simulink model "bee2_calib_corr"  (Simulink 7.1)
settings: Solver=ode45  StartTime=0.0  StopTime=100  RelTol=1e-3
undefined symbols (not defined in the file or in its folder): BEE2_usr
libraries: xbsIndex_r4 x2291, quant_xcorr_lib x1792, xps_library x56, xbsReplacements_r4 x32, casper_library_misc x2
(part 9 of 30)

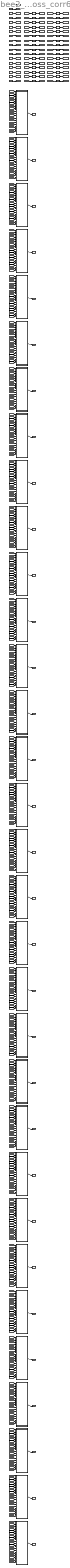
== system bee2_calib_corr/quant_cross_corr6 ==
  rst : Inport  OutDataType=sfix(16)  OutScaling=2^0
  en : Inport  Port=2  OutDataType=sfix(16)  OutScaling=2^0
  in1_16 : Inport  Port=3  OutDataType=sfix(16)  OutScaling=2^0
  in1_17 : Inport  Port=4  OutDataType=sfix(16)  OutScaling=2^0
  in1_18 : Inport  Port=5  OutDataType=sfix(16)  OutScaling=2^0
  in1_19 : Inport  Port=6  OutDataType=sfix(16)  OutScaling=2^0
  in1_20 : Inport  Port=7  OutDataType=sfix(16)  OutScaling=2^0
  in1_21 : Inport  Port=8  OutDataType=sfix(16)  OutScaling=2^0
  in1_22 : Inport  Port=9  OutDataType=sfix(16)  OutScaling=2^0
  in1_23 : Inport  Port=10  OutDataType=sfix(16)  OutScaling=2^0
  in2_16 : Inport  Port=11  OutDataType=sfix(16)  OutScaling=2^0
  in2_17 : Inport  Port=12  OutDataType=sfix(16)  OutScaling=2^0
  in2_18 : Inport  Port=13  OutDataType=sfix(16)  OutScaling=2^0
  in2_19 : Inport  Port=14  OutDataType=sfix(16)  OutScaling=2^0
  in2_20 : Inport  Port=15  OutDataType=sfix(16)  OutScaling=2^0
  in2_21 : Inport  Port=16  OutDataType=sfix(16)  OutScaling=2^0
  in2_22 : Inport  Port=17  OutDataType=sfix(16)  OutScaling=2^0
  in2_23 : Inport  Port=18  OutDataType=sfix(16)  OutScaling=2^0
  in1_16_del1 : xbsIndex_r4/Delay  en=off  latency=1  dbl_ovrd=off  reg_retiming=off  block_type=delay
      settings: xl_area=[0, 0, 0, 0, 0, 0, 0]
  in1_16_del2 : xbsIndex_r4/Delay  en=off  latency=1  dbl_ovrd=off  reg_retiming=off  block_type=delay
      settings: xl_area=[0, 0, 0, 0, 0, 0, 0]
  in1_17_del1 : xbsIndex_r4/Delay  en=off  latency=1  dbl_ovrd=off  reg_retiming=off  block_type=delay
      settings: xl_area=[0, 0, 0, 0, 0, 0, 0]
  in1_17_del2 : xbsIndex_r4/Delay  en=off  latency=1  dbl_ovrd=off  reg_retiming=off  block_type=delay
      settings: xl_area=[0, 0, 0, 0, 0, 0, 0]
  in1_18_del1 : xbsIndex_r4/Delay  en=off  latency=1  dbl_ovrd=off  reg_retiming=off  block_type=delay
      settings: xl_area=[0, 0, 0, 0, 0, 0, 0]
  in1_18_del2 : xbsIndex_r4/Delay  en=off  latency=1  dbl_ovrd=off  reg_retiming=off  block_type=delay
      settings: xl_area=[0, 0, 0, 0, 0, 0, 0]
  in1_19_del1 : xbsIndex_r4/Delay  en=off  latency=1  dbl_ovrd=off  reg_retiming=off  block_type=delay
      settings: xl_area=[0, 0, 0, 0, 0, 0, 0]
  in1_19_del2 : xbsIndex_r4/Delay  en=off  latency=1  dbl_ovrd=off  reg_retiming=off  block_type=delay
      settings: xl_area=[0, 0, 0, 0, 0, 0, 0]
  in1_20_del1 : xbsIndex_r4/Delay  en=off  latency=1  dbl_ovrd=off  reg_retiming=off  block_type=delay
      settings: xl_area=[0, 0, 0, 0, 0, 0, 0]
  in1_20_del2 : xbsIndex_r4/Delay  en=off  latency=1  dbl_ovrd=off  reg_retiming=off  block_type=delay
      settings: xl_area=[0, 0, 0, 0, 0, 0, 0]
  in1_21_del1 : xbsIndex_r4/Delay  en=off  latency=1  dbl_ovrd=off  reg_retiming=off  block_type=delay
      settings: xl_area=[0, 0, 0, 0, 0, 0, 0]
  in1_21_del2 : xbsIndex_r4/Delay  en=off  latency=1  dbl_ovrd=off  reg_retiming=off  block_type=delay
      settings: xl_area=[0, 0, 0, 0, 0, 0, 0]
  in1_22_del1 : xbsIndex_r4/Delay  en=off  latency=1  dbl_ovrd=off  reg_retiming=off  block_type=delay
      settings: xl_area=[0, 0, 0, 0, 0, 0, 0]
  in1_22_del2 : xbsIndex_r4/Delay  en=off  latency=1  dbl_ovrd=off  reg_retiming=off  block_type=delay
      settings: xl_area=[0, 0, 0, 0, 0, 0, 0]
  in1_23_del1 : xbsIndex_r4/Delay  en=off  latency=1  dbl_ovrd=off  reg_retiming=off  block_type=delay
      settings: xl_area=[0, 0, 0, 0, 0, 0, 0]
  in1_23_del2 : xbsIndex_r4/Delay  en=off  latency=1  dbl_ovrd=off  reg_retiming=off  block_type=delay
      settings: xl_area=[0, 0, 0, 0, 0, 0, 0]
  in2_16_del1 : xbsIndex_r4/Delay  en=off  latency=1  dbl_ovrd=off  reg_retiming=off  block_type=delay
      settings: xl_area=[0, 0, 0, 0, 0, 0, 0]
  in2_16_del2 : xbsIndex_r4/Delay  en=off  latency=1  dbl_ovrd=off  reg_retiming=off  block_type=delay
      settings: xl_area=[0, 0, 0, 0, 0, 0, 0]
  in2_17_del1 : xbsIndex_r4/Delay  en=off  latency=1  dbl_ovrd=off  reg_retiming=off  block_type=delay
      settings: xl_area=[0, 0, 0, 0, 0, 0, 0]
  in2_17_del2 : xbsIndex_r4/Delay  en=off  latency=1  dbl_ovrd=off  reg_retiming=off  block_type=delay
      settings: xl_area=[0, 0, 0, 0, 0, 0, 0]
  in2_18_del1 : xbsIndex_r4/Delay  en=off  latency=1  dbl_ovrd=off  reg_retiming=off  block_type=delay
      settings: xl_area=[0, 0, 0, 0, 0, 0, 0]
  in2_18_del2 : xbsIndex_r4/Delay  en=off  latency=1  dbl_ovrd=off  reg_retiming=off  block_type=delay
      settings: xl_area=[0, 0, 0, 0, 0, 0, 0]
  in2_19_del1 : xbsIndex_r4/Delay  en=off  latency=1  dbl_ovrd=off  reg_retiming=off  block_type=delay
      settings: xl_area=[0, 0, 0, 0, 0, 0, 0]
  in2_19_del2 : xbsIndex_r4/Delay  en=off  latency=1  dbl_ovrd=off  reg_retiming=off  block_type=delay
      settings: xl_area=[0, 0, 0, 0, 0, 0, 0]
  in2_20_del1 : xbsIndex_r4/Delay  en=off  latency=1  dbl_ovrd=off  reg_retiming=off  block_type=delay
      settings: xl_area=[0, 0, 0, 0, 0, 0, 0]
  in2_20_del2 : xbsIndex_r4/Delay  en=off  latency=1  dbl_ovrd=off  reg_retiming=off  block_type=delay
      settings: xl_area=[0, 0, 0, 0, 0, 0, 0]
  in2_21_del1 : xbsIndex_r4/Delay  en=off  latency=1  dbl_ovrd=off  reg_retiming=off  block_type=delay
      settings: xl_area=[0, 0, 0, 0, 0, 0, 0]
  in2_21_del2 : xbsIndex_r4/Delay  en=off  latency=1  dbl_ovrd=off  reg_retiming=off  block_type=delay
      settings: xl_area=[0, 0, 0, 0, 0, 0, 0]
  in2_22_del1 : xbsIndex_r4/Delay  en=off  latency=1  dbl_ovrd=off  reg_retiming=off  block_type=delay
      settings: xl_area=[0, 0, 0, 0, 0, 0, 0]
  in2_22_del2 : xbsIndex_r4/Delay  en=off  latency=1  dbl_ovrd=off  reg_retiming=off  block_type=delay
      settings: xl_area=[0, 0, 0, 0, 0, 0, 0]
  in2_23_del1 : xbsIndex_r4/Delay  en=off  latency=1  dbl_ovrd=off  reg_retiming=off  block_type=delay
      settings: xl_area=[0, 0, 0, 0, 0, 0, 0]
  in2_23_del2 : xbsIndex_r4/Delay  en=off  latency=1  dbl_ovrd=off  reg_retiming=off  block_type=delay
      settings: xl_area=[0, 0, 0, 0, 0, 0, 0]
  lag-1 : Subsystem  AncestorBlock=quant_xcorr_lib/quant_lag_element  mask(quant_lag_element){demux=3, lag=-1}
      mask prompts: demux "Demux (2^?):"; lag "Lag Number:"
      mask init:
      | quant_lag_element_init(gcb,...
      |     'demux', demux, 'lag', lag);
      settings: AttributesFormatString=demux=8, lag=-1
  lag-10 : Subsystem  AncestorBlock=quant_xcorr_lib/quant_lag_element  mask(quant_lag_element){demux=3, lag=-10}
      mask prompts: demux "Demux (2^?):"; lag "Lag Number:"
      mask init:
      | quant_lag_element_init(gcb,...
      |     'demux', demux, 'lag', lag);
      settings: AttributesFormatString=demux=8, lag=-10
  lag-11 : Subsystem  AncestorBlock=quant_xcorr_lib/quant_lag_element  mask(quant_lag_element){demux=3, lag=-11}
      mask prompts: demux "Demux (2^?):"; lag "Lag Number:"
      mask init:
      | quant_lag_element_init(gcb,...
      |     'demux', demux, 'lag', lag);
      settings: AttributesFormatString=demux=8, lag=-11
  lag-12 : Subsystem  AncestorBlock=quant_xcorr_lib/quant_lag_element  mask(quant_lag_element){demux=3, lag=-12}
      mask prompts: demux "Demux (2^?):"; lag "Lag Number:"
      mask init:
      | quant_lag_element_init(gcb,...
      |     'demux', demux, 'lag', lag);
      settings: AttributesFormatString=demux=8, lag=-12
  lag-13 : Subsystem  AncestorBlock=quant_xcorr_lib/quant_lag_element  mask(quant_lag_element){demux=3, lag=-13}
      mask prompts: demux "Demux (2^?):"; lag "Lag Number:"
      mask init:
      | quant_lag_element_init(gcb,...
      |     'demux', demux, 'lag', lag);
      settings: AttributesFormatString=demux=8, lag=-13
  lag-14 : Subsystem  AncestorBlock=quant_xcorr_lib/quant_lag_element  mask(quant_lag_element){demux=3, lag=-14}
      mask prompts: demux "Demux (2^?):"; lag "Lag Number:"
      mask init:
      | quant_lag_element_init(gcb,...
      |     'demux', demux, 'lag', lag);
      settings: AttributesFormatString=demux=8, lag=-14
  lag-15 : Subsystem  AncestorBlock=quant_xcorr_lib/quant_lag_element  mask(quant_lag_element){demux=3, lag=-15}
      mask prompts: demux "Demux (2^?):"; lag "Lag Number:"
      mask init:
      | quant_lag_element_init(gcb,...
      |     'demux', demux, 'lag', lag);
      settings: AttributesFormatString=demux=8, lag=-15
  lag-16 : Subsystem  AncestorBlock=quant_xcorr_lib/quant_lag_element  mask(quant_lag_element){demux=3, lag=-16}
      mask prompts: demux "Demux (2^?):"; lag "Lag Number:"
      mask init:
      | quant_lag_element_init(gcb,...
      |     'demux', demux, 'lag', lag);
      settings: AttributesFormatString=demux=8, lag=-16
  lag-2 : Subsystem  AncestorBlock=quant_xcorr_lib/quant_lag_element  mask(quant_lag_element){demux=3, lag=-2}
      mask prompts: demux "Demux (2^?):"; lag "Lag Number:"
      mask init:
      | quant_lag_element_init(gcb,...
      |     'demux', demux, 'lag', lag);
      settings: AttributesFormatString=demux=8, lag=-2
  lag-3 : Subsystem  AncestorBlock=quant_xcorr_lib/quant_lag_element  mask(quant_lag_element){demux=3, lag=-3}
      mask prompts: demux "Demux (2^?):"; lag "Lag Number:"
      mask init:
      | quant_lag_element_init(gcb,...
      |     'demux', demux, 'lag', lag);
      settings: AttributesFormatString=demux=8, lag=-3
  lag-4 : Subsystem  AncestorBlock=quant_xcorr_lib/quant_lag_element  mask(quant_lag_element){demux=3, lag=-4}
      mask prompts: demux "Demux (2^?):"; lag "Lag Number:"
      mask init:
      | quant_lag_element_init(gcb,...
      |     'demux', demux, 'lag', lag);
      settings: AttributesFormatString=demux=8, lag=-4
  lag-5 : Subsystem  AncestorBlock=quant_xcorr_lib/quant_lag_element  mask(quant_lag_element){demux=3, lag=-5}
      mask prompts: demux "Demux (2^?):"; lag "Lag Number:"
      mask init:
      | quant_lag_element_init(gcb,...
      |     'demux', demux, 'lag', lag);
      settings: AttributesFormatString=demux=8, lag=-5
  lag-6 : Subsystem  AncestorBlock=quant_xcorr_lib/quant_lag_element  mask(quant_lag_element){demux=3, lag=-6}
      mask prompts: demux "Demux (2^?):"; lag "Lag Number:"
      mask init:
      | quant_lag_element_init(gcb,...
      |     'demux', demux, 'lag', lag);
      settings: AttributesFormatString=demux=8, lag=-6
  lag-7 : Subsystem  AncestorBlock=quant_xcorr_lib/quant_lag_element  mask(quant_lag_element){demux=3, lag=-7}
      mask prompts: demux "Demux (2^?):"; lag "Lag Number:"
      mask init:
      | quant_lag_element_init(gcb,...
      |     'demux', demux, 'lag', lag);
      settings: AttributesFormatString=demux=8, lag=-7
  lag-8 : Subsystem  AncestorBlock=quant_xcorr_lib/quant_lag_element  mask(quant_lag_element){demux=3, lag=-8}
      mask prompts: demux "Demux (2^?):"; lag "Lag Number:"
      mask init:
      | quant_lag_element_init(gcb,...
      |     'demux', demux, 'lag', lag);
      settings: AttributesFormatString=demux=8, lag=-8
  lag-9 : Subsystem  AncestorBlock=quant_xcorr_lib/quant_lag_element  mask(quant_lag_element){demux=3, lag=-9}
      mask prompts: demux "Demux (2^?):"; lag "Lag Number:"
      mask init:
      | quant_lag_element_init(gcb,...
      |     'demux', demux, 'lag', lag);
      settings: AttributesFormatString=demux=8, lag=-9
  lag0 : Subsystem  AncestorBlock=quant_xcorr_lib/quant_lag_element  mask(quant_lag_element){demux=3, lag=0}
      mask prompts: demux "Demux (2^?):"; lag "Lag Number:"
      mask init:
      | quant_lag_element_init(gcb,...
      |     'demux', demux, 'lag', lag);
      settings: AttributesFormatString=demux=8, lag=0
  lag1 : Subsystem  AncestorBlock=quant_xcorr_lib/quant_lag_element  mask(quant_lag_element){demux=3, lag=1}
      mask prompts: demux "Demux (2^?):"; lag "Lag Number:"
      mask init:
      | quant_lag_element_init(gcb,...
      |     'demux', demux, 'lag', lag);
      settings: AttributesFormatString=demux=8, lag=1
  lag10 : Subsystem  AncestorBlock=quant_xcorr_lib/quant_lag_element  mask(quant_lag_element){demux=3, lag=10}
      mask prompts: demux "Demux (2^?):"; lag "Lag Number:"
      mask init:
      | quant_lag_element_init(gcb,...
      |     'demux', demux, 'lag', lag);
      settings: AttributesFormatString=demux=8, lag=10
  lag11 : Subsystem  AncestorBlock=quant_xcorr_lib/quant_lag_element  mask(quant_lag_element){demux=3, lag=11}
      mask prompts: demux "Demux (2^?):"; lag "Lag Number:"
      mask init:
      | quant_lag_element_init(gcb,...
      |     'demux', demux, 'lag', lag);
      settings: AttributesFormatString=demux=8, lag=11
  lag12 : Subsystem  AncestorBlock=quant_xcorr_lib/quant_lag_element  mask(quant_lag_element){demux=3, lag=12}
      mask prompts: demux "Demux (2^?):"; lag "Lag Number:"
      mask init:
      | quant_lag_element_init(gcb,...
      |     'demux', demux, 'lag', lag);
      settings: AttributesFormatString=demux=8, lag=12
  lag13 : Subsystem  AncestorBlock=quant_xcorr_lib/quant_lag_element  mask(quant_lag_element){demux=3, lag=13}
      mask prompts: demux "Demux (2^?):"; lag "Lag Number:"
      mask init:
      | quant_lag_element_init(gcb,...
      |     'demux', demux, 'lag', lag);
      settings: AttributesFormatString=demux=8, lag=13
  lag14 : Subsystem  AncestorBlock=quant_xcorr_lib/quant_lag_element  mask(quant_lag_element){demux=3, lag=14}
      mask prompts: demux "Demux (2^?):"; lag "Lag Number:"
      mask init:
      | quant_lag_element_init(gcb,...
      |     'demux', demux, 'lag', lag);
      settings: AttributesFormatString=demux=8, lag=14
  lag15 : Subsystem  AncestorBlock=quant_xcorr_lib/quant_lag_element  mask(quant_lag_element){demux=3, lag=15}
      mask prompts: demux "Demux (2^?):"; lag "Lag Number:"
      mask init:
      | quant_lag_element_init(gcb,...
      |     'demux', demux, 'lag', lag);
      settings: AttributesFormatString=demux=8, lag=15
  lag2 : Subsystem  AncestorBlock=quant_xcorr_lib/quant_lag_element  mask(quant_lag_element){demux=3, lag=2}
      mask prompts: demux "Demux (2^?):"; lag "Lag Number:"
      mask init:
      | quant_lag_element_init(gcb,...
      |     'demux', demux, 'lag', lag);
      settings: AttributesFormatString=demux=8, lag=2
  lag3 : Subsystem  AncestorBlock=quant_xcorr_lib/quant_lag_element  mask(quant_lag_element){demux=3, lag=3}
      mask prompts: demux "Demux (2^?):"; lag "Lag Number:"
      mask init:
      | quant_lag_element_init(gcb,...
      |     'demux', demux, 'lag', lag);
      settings: AttributesFormatString=demux=8, lag=3
  lag4 : Subsystem  AncestorBlock=quant_xcorr_lib/quant_lag_element  mask(quant_lag_element){demux=3, lag=4}
      mask prompts: demux "Demux (2^?):"; lag "Lag Number:"
      mask init:
      | quant_lag_element_init(gcb,...
      |     'demux', demux, 'lag', lag);
      settings: AttributesFormatString=demux=8, lag=4
  lag5 : Subsystem  AncestorBlock=quant_xcorr_lib/quant_lag_element  mask(quant_lag_element){demux=3, lag=5}
      mask prompts: demux "Demux (2^?):"; lag "Lag Number:"
      mask init:
      | quant_lag_element_init(gcb,...
      |     'demux', demux, 'lag', lag);
      settings: AttributesFormatString=demux=8, lag=5
  lag6 : Subsystem  AncestorBlock=quant_xcorr_lib/quant_lag_element  mask(quant_lag_element){demux=3, lag=6}
      mask prompts: demux "Demux (2^?):"; lag "Lag Number:"
      mask init:
      | quant_lag_element_init(gcb,...
      |     'demux', demux, 'lag', lag);
      settings: AttributesFormatString=demux=8, lag=6
  lag7 : Subsystem  AncestorBlock=quant_xcorr_lib/quant_lag_element  mask(quant_lag_element){demux=3, lag=7}
      mask prompts: demux "Demux (2^?):"; lag "Lag Number:"
      mask init:
      | quant_lag_element_init(gcb,...
      |     'demux', demux, 'lag', lag);
      settings: AttributesFormatString=demux=8, lag=7
  lag8 : Subsystem  AncestorBlock=quant_xcorr_lib/quant_lag_element  mask(quant_lag_element){demux=3, lag=8}
      mask prompts: demux "Demux (2^?):"; lag "Lag Number:"
      mask init:
      | quant_lag_element_init(gcb,...
      |     'demux', demux, 'lag', lag);
      settings: AttributesFormatString=demux=8, lag=8
  lag9 : Subsystem  AncestorBlock=quant_xcorr_lib/quant_lag_element  mask(quant_lag_element){demux=3, lag=9}
      mask prompts: demux "Demux (2^?):"; lag "Lag Number:"
      mask init:
      | quant_lag_element_init(gcb,...
      |     'demux', demux, 'lag', lag);
      settings: AttributesFormatString=demux=8, lag=9
  out-16 : Outport  OutDataType=sfix(16)  OutScaling=2^0
  out-15 : Outport  Port=2  OutDataType=sfix(16)  OutScaling=2^0
  out-14 : Outport  Port=3  OutDataType=sfix(16)  OutScaling=2^0
  out-13 : Outport  Port=4  OutDataType=sfix(16)  OutScaling=2^0
  out-12 : Outport  Port=5  OutDataType=sfix(16)  OutScaling=2^0
  out-11 : Outport  Port=6  OutDataType=sfix(16)  OutScaling=2^0
  out-10 : Outport  Port=7  OutDataType=sfix(16)  OutScaling=2^0
  out-9 : Outport  Port=8  OutDataType=sfix(16)  OutScaling=2^0
  out-8 : Outport  Port=9  OutDataType=sfix(16)  OutScaling=2^0
  out-7 : Outport  Port=10  OutDataType=sfix(16)  OutScaling=2^0
  out-6 : Outport  Port=11  OutDataType=sfix(16)  OutScaling=2^0
  out-5 : Outport  Port=12  OutDataType=sfix(16)  OutScaling=2^0
  out-4 : Outport  Port=13  OutDataType=sfix(16)  OutScaling=2^0
  out-3 : Outport  Port=14  OutDataType=sfix(16)  OutScaling=2^0
  out-2 : Outport  Port=15  OutDataType=sfix(16)  OutScaling=2^0
  out-1 : Outport  Port=16  OutDataType=sfix(16)  OutScaling=2^0
  out0 : Outport  Port=17  OutDataType=sfix(16)  OutScaling=2^0
  out1 : Outport  Port=18  OutDataType=sfix(16)  OutScaling=2^0
  out2 : Outport  Port=19  OutDataType=sfix(16)  OutScaling=2^0
  out3 : Outport  Port=20  OutDataType=sfix(16)  OutScaling=2^0
  out4 : Outport  Port=21  OutDataType=sfix(16)  OutScaling=2^0
  out5 : Outport  Port=22  OutDataType=sfix(16)  OutScaling=2^0
  out6 : Outport  Port=23  OutDataType=sfix(16)  OutScaling=2^0
  out7 : Outport  Port=24  OutDataType=sfix(16)  OutScaling=2^0
  out8 : Outport  Port=25  OutDataType=sfix(16)  OutScaling=2^0
  out9 : Outport  Port=26  OutDataType=sfix(16)  OutScaling=2^0
  out10 : Outport  Port=27  OutDataType=sfix(16)  OutScaling=2^0
  out11 : Outport  Port=28  OutDataType=sfix(16)  OutScaling=2^0
  out12 : Outport  Port=29  OutDataType=sfix(16)  OutScaling=2^0
  out13 : Outport  Port=30  OutDataType=sfix(16)  OutScaling=2^0
  out14 : Outport  Port=31  OutDataType=sfix(16)  OutScaling=2^0
  out15 : Outport  Port=32  OutDataType=sfix(16)  OutScaling=2^0
  wires:
  rst/1 -> [reset]
  en/1 -> [enable]
  in1_16/1 -> [in1_16]
  [in1_16] -> in1_16_del1/1
  in1_16_del1/1 -> [in1_8]
  [in1_8] -> in1_16_del2/1
  in1_16_del2/1 -> [in1_0]
  in1_17/1 -> [in1_17]
  [in1_17] -> in1_17_del1/1
  in1_17_del1/1 -> [in1_9]
  [in1_9] -> in1_17_del2/1
  in1_17_del2/1 -> [in1_1]
  in1_18/1 -> [in1_18]
  [in1_18] -> in1_18_del1/1
  in1_18_del1/1 -> [in1_10]
  [in1_10] -> in1_18_del2/1
  in1_18_del2/1 -> [in1_2]
  in1_19/1 -> [in1_19]
  [in1_19] -> in1_19_del1/1
  in1_19_del1/1 -> [in1_11]
  [in1_11] -> in1_19_del2/1
  in1_19_del2/1 -> [in1_3]
  in1_20/1 -> [in1_20]
  [in1_20] -> in1_20_del1/1
  in1_20_del1/1 -> [in1_12]
  [in1_12] -> in1_20_del2/1
  in1_20_del2/1 -> [in1_4]
  in1_21/1 -> [in1_21]
  [in1_21] -> in1_21_del1/1
  in1_21_del1/1 -> [in1_13]
  [in1_13] -> in1_21_del2/1
  in1_21_del2/1 -> [in1_5]
  in1_22/1 -> [in1_22]
  [in1_22] -> in1_22_del1/1
  in1_22_del1/1 -> [in1_14]
  [in1_14] -> in1_22_del2/1
  in1_22_del2/1 -> [in1_6]
  in1_23/1 -> [in1_23]
  [in1_23] -> in1_23_del1/1
  in1_23_del1/1 -> [in1_15]
  [in1_15] -> in1_23_del2/1
  in1_23_del2/1 -> [in1_7]
  in2_16/1 -> [in2_16]
  [in2_16] -> in2_16_del1/1
  in2_16_del1/1 -> [in2_8]
  [in2_8] -> in2_16_del2/1
  in2_16_del2/1 -> [in2_0]
  in2_17/1 -> [in2_17]
  [in2_17] -> in2_17_del1/1
  in2_17_del1/1 -> [in2_9]
  [in2_9] -> in2_17_del2/1
  in2_17_del2/1 -> [in2_1]
  in2_18/1 -> [in2_18]
  [in2_18] -> in2_18_del1/1
  in2_18_del1/1 -> [in2_10]
  [in2_10] -> in2_18_del2/1
  in2_18_del2/1 -> [in2_2]
  in2_19/1 -> [in2_19]
  [in2_19] -> in2_19_del1/1
  in2_19_del1/1 -> [in2_11]
  [in2_11] -> in2_19_del2/1
  in2_19_del2/1 -> [in2_3]
  in2_20/1 -> [in2_20]
  [in2_20] -> in2_20_del1/1
  in2_20_del1/1 -> [in2_12]
  [in2_12] -> in2_20_del2/1
  in2_20_del2/1 -> [in2_4]
  in2_21/1 -> [in2_21]
  [in2_21] -> in2_21_del1/1
  in2_21_del1/1 -> [in2_13]
  [in2_13] -> in2_21_del2/1
  in2_21_del2/1 -> [in2_5]
  in2_22/1 -> [in2_22]
  [in2_22] -> in2_22_del1/1
  in2_22_del1/1 -> [in2_14]
  [in2_14] -> in2_22_del2/1
  in2_22_del2/1 -> [in2_6]
  in2_23/1 -> [in2_23]
  [in2_23] -> in2_23_del1/1
  in2_23_del1/1 -> [in2_15]
  [in2_15] -> in2_23_del2/1
  in2_23_del2/1 -> [in2_7]
  [in1_16] -> lag-16/1(in1_0)
  [in1_17] -> lag-16/2(in1_1)
  [in1_18] -> lag-16/3(in1_2)
  [in1_19] -> lag-16/4(in1_3)
  [in1_20] -> lag-16/5(in1_4)
  [in1_21] -> lag-16/6(in1_5)
  [in1_22] -> lag-16/7(in1_6)
  [in1_23] -> lag-16/8(in1_7)
  [in2_0] -> lag-16/9(in2_0)
  [in2_1] -> lag-16/10(in2_1)
  [in2_2] -> lag-16/11(in2_2)
  [in2_3] -> lag-16/12(in2_3)
  [in2_4] -> lag-16/13(in2_4)
  [in2_5] -> lag-16/14(in2_5)
  [in2_6] -> lag-16/15(in2_6)
  [in2_7] -> lag-16/16(in2_7)
  [reset] -> lag-16/17(rst)
  [enable] -> lag-16/18(en)
  lag-16/1(acc_out) -> out-16/1
  [in1_15] -> lag-15/1(in1_0)
  [in1_16] -> lag-15/2(in1_1)
  [in1_17] -> lag-15/3(in1_2)
  [in1_18] -> lag-15/4(in1_3)
  [in1_19] -> lag-15/5(in1_4)
  [in1_20] -> lag-15/6(in1_5)
  [in1_21] -> lag-15/7(in1_6)
  [in1_22] -> lag-15/8(in1_7)
  [in2_0] -> lag-15/9(in2_0)
  [in2_1] -> lag-15/10(in2_1)
  [in2_2] -> lag-15/11(in2_2)
  [in2_3] -> lag-15/12(in2_3)
  [in2_4] -> lag-15/13(in2_4)
  [in2_5] -> lag-15/14(in2_5)
  [in2_6] -> lag-15/15(in2_6)
  [in2_7] -> lag-15/16(in2_7)
  [reset] -> lag-15/17(rst)
  [enable] -> lag-15/18(en)
  lag-15/1(acc_out) -> out-15/1
  [in1_14] -> lag-14/1(in1_0)
  [in1_15] -> lag-14/2(in1_1)
  [in1_16] -> lag-14/3(in1_2)
  [in1_17] -> lag-14/4(in1_3)
  [in1_18] -> lag-14/5(in1_4)
  [in1_19] -> lag-14/6(in1_5)
  [in1_20] -> lag-14/7(in1_6)
  [in1_21] -> lag-14/8(in1_7)
  [in2_0] -> lag-14/9(in2_0)
  [in2_1] -> lag-14/10(in2_1)
  [in2_2] -> lag-14/11(in2_2)
  [in2_3] -> lag-14/12(in2_3)
  [in2_4] -> lag-14/13(in2_4)
  [in2_5] -> lag-14/14(in2_5)
  [in2_6] -> lag-14/15(in2_6)
  [in2_7] -> lag-14/16(in2_7)
  [reset] -> lag-14/17(rst)
  [enable] -> lag-14/18(en)
  lag-14/1(acc_out) -> out-14/1
  [in1_13] -> lag-13/1(in1_0)
  [in1_14] -> lag-13/2(in1_1)
  [in1_15] -> lag-13/3(in1_2)
  [in1_16] -> lag-13/4(in1_3)
  [in1_17] -> lag-13/5(in1_4)
  [in1_18] -> lag-13/6(in1_5)
  [in1_19] -> lag-13/7(in1_6)
  [in1_20] -> lag-13/8(in1_7)
  [in2_0] -> lag-13/9(in2_0)
  [in2_1] -> lag-13/10(in2_1)
  [in2_2] -> lag-13/11(in2_2)
  [in2_3] -> lag-13/12(in2_3)
  [in2_4] -> lag-13/13(in2_4)
  [in2_5] -> lag-13/14(in2_5)
  [in2_6] -> lag-13/15(in2_6)
  [in2_7] -> lag-13/16(in2_7)
  [reset] -> lag-13/17(rst)
  [enable] -> lag-13/18(en)
  lag-13/1(acc_out) -> out-13/1
  [in1_12] -> lag-12/1(in1_0)
  [in1_13] -> lag-12/2(in1_1)
  [in1_14] -> lag-12/3(in1_2)
  [in1_15] -> lag-12/4(in1_3)
  [in1_16] -> lag-12/5(in1_4)
  [in1_17] -> lag-12/6(in1_5)
  [in1_18] -> lag-12/7(in1_6)
  [in1_19] -> lag-12/8(in1_7)
  [in2_0] -> lag-12/9(in2_0)
  [in2_1] -> lag-12/10(in2_1)
  [in2_2] -> lag-12/11(in2_2)
  [in2_3] -> lag-12/12(in2_3)
  [in2_4] -> lag-12/13(in2_4)
  [in2_5] -> lag-12/14(in2_5)
  [in2_6] -> lag-12/15(in2_6)
  [in2_7] -> lag-12/16(in2_7)
  [reset] -> lag-12/17(rst)
  [enable] -> lag-12/18(en)
  lag-12/1(acc_out) -> out-12/1
  [in1_11] -> lag-11/1(in1_0)
  [in1_12] -> lag-11/2(in1_1)
  [in1_13] -> lag-11/3(in1_2)
  [in1_14] -> lag-11/4(in1_3)
  [in1_15] -> lag-11/5(in1_4)
  [in1_16] -> lag-11/6(in1_5)
  [in1_17] -> lag-11/7(in1_6)
  [in1_18] -> lag-11/8(in1_7)
  [in2_0] -> lag-11/9(in2_0)
  [in2_1] -> lag-11/10(in2_1)
  [in2_2] -> lag-11/11(in2_2)
  [in2_3] -> lag-11/12(in2_3)
  [in2_4] -> lag-11/13(in2_4)
  [in2_5] -> lag-11/14(in2_5)
  [in2_6] -> lag-11/15(in2_6)
  [in2_7] -> lag-11/16(in2_7)
  [reset] -> lag-11/17(rst)
  [enable] -> lag-11/18(en)
  lag-11/1(acc_out) -> out-11/1
  [in1_10] -> lag-10/1(in1_0)
  [in1_11] -> lag-10/2(in1_1)
  [in1_12] -> lag-10/3(in1_2)
  [in1_13] -> lag-10/4(in1_3)
  [in1_14] -> lag-10/5(in1_4)
  [in1_15] -> lag-10/6(in1_5)
  [in1_16] -> lag-10/7(in1_6)
  [in1_17] -> lag-10/8(in1_7)
  [in2_0] -> lag-10/9(in2_0)
  [in2_1] -> lag-10/10(in2_1)
  [in2_2] -> lag-10/11(in2_2)
  [in2_3] -> lag-10/12(in2_3)
  [in2_4] -> lag-10/13(in2_4)
  [in2_5] -> lag-10/14(in2_5)
  [in2_6] -> lag-10/15(in2_6)
  [in2_7] -> lag-10/16(in2_7)
  [reset] -> lag-10/17(rst)
  [enable] -> lag-10/18(en)
  lag-10/1(acc_out) -> out-10/1
  [in1_9] -> lag-9/1(in1_0)
  [in1_10] -> lag-9/2(in1_1)
  [in1_11] -> lag-9/3(in1_2)
  [in1_12] -> lag-9/4(in1_3)
  [in1_13] -> lag-9/5(in1_4)
  [in1_14] -> lag-9/6(in1_5)
  [in1_15] -> lag-9/7(in1_6)
  [in1_16] -> lag-9/8(in1_7)
  [in2_0] -> lag-9/9(in2_0)
  [in2_1] -> lag-9/10(in2_1)
  [in2_2] -> lag-9/11(in2_2)
  [in2_3] -> lag-9/12(in2_3)
  [in2_4] -> lag-9/13(in2_4)
  [in2_5] -> lag-9/14(in2_5)
  [in2_6] -> lag-9/15(in2_6)
  [in2_7] -> lag-9/16(in2_7)
  [reset] -> lag-9/17(rst)
  [enable] -> lag-9/18(en)
  lag-9/1(acc_out) -> out-9/1
  [in1_8] -> lag-8/1(in1_0)
  [in1_9] -> lag-8/2(in1_1)
  [in1_10] -> lag-8/3(in1_2)
  [in1_11] -> lag-8/4(in1_3)
  [in1_12] -> lag-8/5(in1_4)
  [in1_13] -> lag-8/6(in1_5)
  [in1_14] -> lag-8/7(in1_6)
  [in1_15] -> lag-8/8(in1_7)
  [in2_0] -> lag-8/9(in2_0)
  [in2_1] -> lag-8/10(in2_1)
  [in2_2] -> lag-8/11(in2_2)
  [in2_3] -> lag-8/12(in2_3)
  [in2_4] -> lag-8/13(in2_4)
  [in2_5] -> lag-8/14(in2_5)
  [in2_6] -> lag-8/15(in2_6)
  [in2_7] -> lag-8/16(in2_7)
  [reset] -> lag-8/17(rst)
  [enable] -> lag-8/18(en)
  lag-8/1(acc_out) -> out-8/1
  [in1_7] -> lag-7/1(in1_0)
  [in1_8] -> lag-7/2(in1_1)
  [in1_9] -> lag-7/3(in1_2)
  [in1_10] -> lag-7/4(in1_3)
  [in1_11] -> lag-7/5(in1_4)
  [in1_12] -> lag-7/6(in1_5)
  [in1_13] -> lag-7/7(in1_6)
  [in1_14] -> lag-7/8(in1_7)
  [in2_0] -> lag-7/9(in2_0)
  [in2_1] -> lag-7/10(in2_1)
  [in2_2] -> lag-7/11(in2_2)
  [in2_3] -> lag-7/12(in2_3)
  [in2_4] -> lag-7/13(in2_4)
  [in2_5] -> lag-7/14(in2_5)
  [in2_6] -> lag-7/15(in2_6)
  [in2_7] -> lag-7/16(in2_7)
  [reset] -> lag-7/17(rst)
  [enable] -> lag-7/18(en)
  lag-7/1(acc_out) -> out-7/1
  [in1_6] -> lag-6/1(in1_0)
  [in1_7] -> lag-6/2(in1_1)
  [in1_8] -> lag-6/3(in1_2)
  [in1_9] -> lag-6/4(in1_3)
  [in1_10] -> lag-6/5(in1_4)
  [in1_11] -> lag-6/6(in1_5)
  [in1_12] -> lag-6/7(in1_6)
  [in1_13] -> lag-6/8(in1_7)
  [in2_0] -> lag-6/9(in2_0)
  [in2_1] -> lag-6/10(in2_1)
  [in2_2] -> lag-6/11(in2_2)
  [in2_3] -> lag-6/12(in2_3)
  [in2_4] -> lag-6/13(in2_4)
  [in2_5] -> lag-6/14(in2_5)
  [in2_6] -> lag-6/15(in2_6)
  [in2_7] -> lag-6/16(in2_7)
  [reset] -> lag-6/17(rst)
  [enable] -> lag-6/18(en)
  lag-6/1(acc_out) -> out-6/1
  [in1_5] -> lag-5/1(in1_0)
  [in1_6] -> lag-5/2(in1_1)
  [in1_7] -> lag-5/3(in1_2)
  [in1_8] -> lag-5/4(in1_3)
  [in1_9] -> lag-5/5(in1_4)
  [in1_10] -> lag-5/6(in1_5)
  [in1_11] -> lag-5/7(in1_6)
  [in1_12] -> lag-5/8(in1_7)
  [in2_0] -> lag-5/9(in2_0)
  [in2_1] -> lag-5/10(in2_1)
  [in2_2] -> lag-5/11(in2_2)
  [in2_3] -> lag-5/12(in2_3)
  [in2_4] -> lag-5/13(in2_4)
  [in2_5] -> lag-5/14(in2_5)
  [in2_6] -> lag-5/15(in2_6)
  [in2_7] -> lag-5/16(in2_7)
  [reset] -> lag-5/17(rst)
  [enable] -> lag-5/18(en)
  lag-5/1(acc_out) -> out-5/1
  [in1_4] -> lag-4/1(in1_0)
  [in1_5] -> lag-4/2(in1_1)
  [in1_6] -> lag-4/3(in1_2)
  [in1_7] -> lag-4/4(in1_3)
  [in1_8] -> lag-4/5(in1_4)
  [in1_9] -> lag-4/6(in1_5)
  [in1_10] -> lag-4/7(in1_6)
  [in1_11] -> lag-4/8(in1_7)
  [in2_0] -> lag-4/9(in2_0)
  [in2_1] -> lag-4/10(in2_1)
  [in2_2] -> lag-4/11(in2_2)
  [in2_3] -> lag-4/12(in2_3)
  [in2_4] -> lag-4/13(in2_4)
  [in2_5] -> lag-4/14(in2_5)
  [in2_6] -> lag-4/15(in2_6)
  [in2_7] -> lag-4/16(in2_7)
  [reset] -> lag-4/17(rst)
  [enable] -> lag-4/18(en)
  lag-4/1(acc_out) -> out-4/1
  [in1_3] -> lag-3/1(in1_0)
  [in1_4] -> lag-3/2(in1_1)
  [in1_5] -> lag-3/3(in1_2)
  [in1_6] -> lag-3/4(in1_3)
  [in1_7] -> lag-3/5(in1_4)
  [in1_8] -> lag-3/6(in1_5)
  [in1_9] -> lag-3/7(in1_6)
  [in1_10] -> lag-3/8(in1_7)
  [in2_0] -> lag-3/9(in2_0)
  [in2_1] -> lag-3/10(in2_1)
  [in2_2] -> lag-3/11(in2_2)
  [in2_3] -> lag-3/12(in2_3)
  [in2_4] -> lag-3/13(in2_4)
  [in2_5] -> lag-3/14(in2_5)
  [in2_6] -> lag-3/15(in2_6)
  [in2_7] -> lag-3/16(in2_7)
  [reset] -> lag-3/17(rst)
  [enable] -> lag-3/18(en)
  lag-3/1(acc_out) -> out-3/1
  [in1_2] -> lag-2/1(in1_0)
  [in1_3] -> lag-2/2(in1_1)
  [in1_4] -> lag-2/3(in1_2)
  [in1_5] -> lag-2/4(in1_3)
  [in1_6] -> lag-2/5(in1_4)
  [in1_7] -> lag-2/6(in1_5)
  [in1_8] -> lag-2/7(in1_6)
  [in1_9] -> lag-2/8(in1_7)
  [in2_0] -> lag-2/9(in2_0)
  [in2_1] -> lag-2/10(in2_1)
  [in2_2] -> lag-2/11(in2_2)
  [in2_3] -> lag-2/12(in2_3)
  [in2_4] -> lag-2/13(in2_4)
  [in2_5] -> lag-2/14(in2_5)
  [in2_6] -> lag-2/15(in2_6)
  [in2_7] -> lag-2/16(in2_7)
  [reset] -> lag-2/17(rst)
  [enable] -> lag-2/18(en)
  lag-2/1(acc_out) -> out-2/1
  [in1_1] -> lag-1/1(in1_0)
  [in1_2] -> lag-1/2(in1_1)
  [in1_3] -> lag-1/3(in1_2)
  [in1_4] -> lag-1/4(in1_3)
  [in1_5] -> lag-1/5(in1_4)
  [in1_6] -> lag-1/6(in1_5)
  [in1_7] -> lag-1/7(in1_6)
  [in1_8] -> lag-1/8(in1_7)
  [in2_0] -> lag-1/9(in2_0)
  [in2_1] -> lag-1/10(in2_1)
  [in2_2] -> lag-1/11(in2_2)
  [in2_3] -> lag-1/12(in2_3)
  [in2_4] -> lag-1/13(in2_4)
  [in2_5] -> lag-1/14(in2_5)
  [in2_6] -> lag-1/15(in2_6)
  [in2_7] -> lag-1/16(in2_7)
  [reset] -> lag-1/17(rst)
  [enable] -> lag-1/18(en)
  lag-1/1(acc_out) -> out-1/1
  [in1_0] -> lag0/1(in1_0)
  [in1_1] -> lag0/2(in1_1)
  [in1_2] -> lag0/3(in1_2)
  [in1_3] -> lag0/4(in1_3)
  [in1_4] -> lag0/5(in1_4)
  [in1_5] -> lag0/6(in1_5)
  [in1_6] -> lag0/7(in1_6)
  [in1_7] -> lag0/8(in1_7)
  [in2_0] -> lag0/9(in2_0)
  [in2_1] -> lag0/10(in2_1)
  [in2_2] -> lag0/11(in2_2)
  [in2_3] -> lag0/12(in2_3)
  [in2_4] -> lag0/13(in2_4)
  [in2_5] -> lag0/14(in2_5)
  [in2_6] -> lag0/15(in2_6)
  [in2_7] -> lag0/16(in2_7)
  [reset] -> lag0/17(rst)
  [enable] -> lag0/18(en)
  lag0/1(acc_out) -> out0/1
  [in1_0] -> lag1/1(in1_0)
  [in1_1] -> lag1/2(in1_1)
  [in1_2] -> lag1/3(in1_2)
  [in1_3] -> lag1/4(in1_3)
  [in1_4] -> lag1/5(in1_4)
  [in1_5] -> lag1/6(in1_5)
  [in1_6] -> lag1/7(in1_6)
  [in1_7] -> lag1/8(in1_7)
  [in2_1] -> lag1/9(in2_0)
  [in2_2] -> lag1/10(in2_1)
  [in2_3] -> lag1/11(in2_2)
  [in2_4] -> lag1/12(in2_3)
  [in2_5] -> lag1/13(in2_4)
  [in2_6] -> lag1/14(in2_5)
  [in2_7] -> lag1/15(in2_6)
  [in2_8] -> lag1/16(in2_7)
  [reset] -> lag1/17(rst)
  [enable] -> lag1/18(en)
  lag1/1(acc_out) -> out1/1
  [in1_0] -> lag2/1(in1_0)
  [in1_1] -> lag2/2(in1_1)
  [in1_2] -> lag2/3(in1_2)
  [in1_3] -> lag2/4(in1_3)
  [in1_4] -> lag2/5(in1_4)
  [in1_5] -> lag2/6(in1_5)
  [in1_6] -> lag2/7(in1_6)
  [in1_7] -> lag2/8(in1_7)
  [in2_2] -> lag2/9(in2_0)
  [in2_3] -> lag2/10(in2_1)
  [in2_4] -> lag2/11(in2_2)
  [in2_5] -> lag2/12(in2_3)
  [in2_6] -> lag2/13(in2_4)
  [in2_7] -> lag2/14(in2_5)
  [in2_8] -> lag2/15(in2_6)
  [in2_9] -> lag2/16(in2_7)
  [reset] -> lag2/17(rst)
  [enable] -> lag2/18(en)
  lag2/1(acc_out) -> out2/1
  [in1_0] -> lag3/1(in1_0)
  [in1_1] -> lag3/2(in1_1)
  [in1_2] -> lag3/3(in1_2)
  [in1_3] -> lag3/4(in1_3)
  [in1_4] -> lag3/5(in1_4)
  [in1_5] -> lag3/6(in1_5)
  [in1_6] -> lag3/7(in1_6)
  [in1_7] -> lag3/8(in1_7)
  [in2_3] -> lag3/9(in2_0)
  [in2_4] -> lag3/10(in2_1)
  [in2_5] -> lag3/11(in2_2)
  [in2_6] -> lag3/12(in2_3)
  [in2_7] -> lag3/13(in2_4)
  [in2_8] -> lag3/14(in2_5)
  [in2_9] -> lag3/15(in2_6)
  [in2_10] -> lag3/16(in2_7)
  [reset] -> lag3/17(rst)
  [enable] -> lag3/18(en)
  lag3/1(acc_out) -> out3/1
  [in1_0] -> lag4/1(in1_0)
  [in1_1] -> lag4/2(in1_1)
  [in1_2] -> lag4/3(in1_2)
  [in1_3] -> lag4/4(in1_3)
  [in1_4] -> lag4/5(in1_4)
  [in1_5] -> lag4/6(in1_5)
  [in1_6] -> lag4/7(in1_6)
  [in1_7] -> lag4/8(in1_7)
  [in2_4] -> lag4/9(in2_0)
  [in2_5] -> lag4/10(in2_1)
  [in2_6] -> lag4/11(in2_2)
  [in2_7] -> lag4/12(in2_3)
  [in2_8] -> lag4/13(in2_4)
  [in2_9] -> lag4/14(in2_5)
  [in2_10] -> lag4/15(in2_6)
  [in2_11] -> lag4/16(in2_7)
  [reset] -> lag4/17(rst)
  [enable] -> lag4/18(en)
  lag4/1(acc_out) -> out4/1
  [in1_0] -> lag5/1(in1_0)
  [in1_1] -> lag5/2(in1_1)
  [in1_2] -> lag5/3(in1_2)
  [in1_3] -> lag5/4(in1_3)
  [in1_4] -> lag5/5(in1_4)
  [in1_5] -> lag5/6(in1_5)
  [in1_6] -> lag5/7(in1_6)
  [in1_7] -> lag5/8(in1_7)
  [in2_5] -> lag5/9(in2_0)
  [in2_6] -> lag5/10(in2_1)
  [in2_7] -> lag5/11(in2_2)
  [in2_8] -> lag5/12(in2_3)
  [in2_9] -> lag5/13(in2_4)
  [in2_10] -> lag5/14(in2_5)
  [in2_11] -> lag5/15(in2_6)
  [in2_12] -> lag5/16(in2_7)
  [reset] -> lag5/17(rst)
  [enable] -> lag5/18(en)
  lag5/1(acc_out) -> out5/1
  [in1_0] -> lag6/1(in1_0)
  [in1_1] -> lag6/2(in1_1)
  [in1_2] -> lag6/3(in1_2)
  [in1_3] -> lag6/4(in1_3)
  [in1_4] -> lag6/5(in1_4)
  [in1_5] -> lag6/6(in1_5)
  [in1_6] -> lag6/7(in1_6)
  [in1_7] -> lag6/8(in1_7)
  [in2_6] -> lag6/9(in2_0)
  [in2_7] -> lag6/10(in2_1)
  [in2_8] -> lag6/11(in2_2)
  [in2_9] -> lag6/12(in2_3)
  [in2_10] -> lag6/13(in2_4)
  [in2_11] -> lag6/14(in2_5)
  [in2_12] -> lag6/15(in2_6)
  [in2_13] -> lag6/16(in2_7)
  [reset] -> lag6/17(rst)
  [enable] -> lag6/18(en)
  lag6/1(acc_out) -> out6/1
  [in1_0] -> lag7/1(in1_0)
  [in1_1] -> lag7/2(in1_1)
  [in1_2] -> lag7/3(in1_2)
  [in1_3] -> lag7/4(in1_3)
  [in1_4] -> lag7/5(in1_4)
  [in1_5] -> lag7/6(in1_5)
  [in1_6] -> lag7/7(in1_6)
  [in1_7] -> lag7/8(in1_7)
  [in2_7] -> lag7/9(in2_0)
  [in2_8] -> lag7/10(in2_1)
  [in2_9] -> lag7/11(in2_2)
  [in2_10] -> lag7/12(in2_3)
  [in2_11] -> lag7/13(in2_4)
  [in2_12] -> lag7/14(in2_5)
  [in2_13] -> lag7/15(in2_6)
  [in2_14] -> lag7/16(in2_7)
  [reset] -> lag7/17(rst)
  [enable] -> lag7/18(en)
  lag7/1(acc_out) -> out7/1
  [in1_0] -> lag8/1(in1_0)
  [in1_1] -> lag8/2(in1_1)
  [in1_2] -> lag8/3(in1_2)
  [in1_3] -> lag8/4(in1_3)
  [in1_4] -> lag8/5(in1_4)
  [in1_5] -> lag8/6(in1_5)
  [in1_6] -> lag8/7(in1_6)
  [in1_7] -> lag8/8(in1_7)
  [in2_8] -> lag8/9(in2_0)
  [in2_9] -> lag8/10(in2_1)
  [in2_10] -> lag8/11(in2_2)
  [in2_11] -> lag8/12(in2_3)
  [in2_12] -> lag8/13(in2_4)
  [in2_13] -> lag8/14(in2_5)
  [in2_14] -> lag8/15(in2_6)
  [in2_15] -> lag8/16(in2_7)
  [reset] -> lag8/17(rst)
  [enable] -> lag8/18(en)
  lag8/1(acc_out) -> out8/1
  [in1_0] -> lag9/1(in1_0)
  [in1_1] -> lag9/2(in1_1)
  [in1_2] -> lag9/3(in1_2)
  [in1_3] -> lag9/4(in1_3)
  [in1_4] -> lag9/5(in1_4)
  [in1_5] -> lag9/6(in1_5)
  [in1_6] -> lag9/7(in1_6)
  [in1_7] -> lag9/8(in1_7)
  [in2_9] -> lag9/9(in2_0)
  [in2_10] -> lag9/10(in2_1)
  [in2_11] -> lag9/11(in2_2)
  [in2_12] -> lag9/12(in2_3)
  [in2_13] -> lag9/13(in2_4)
  [in2_14] -> lag9/14(in2_5)
  [in2_15] -> lag9/15(in2_6)
  [in2_16] -> lag9/16(in2_7)
  [reset] -> lag9/17(rst)
  [enable] -> lag9/18(en)
  lag9/1(acc_out) -> out9/1
  [in1_0] -> lag10/1(in1_0)
  [in1_1] -> lag10/2(in1_1)
  [in1_2] -> lag10/3(in1_2)
  [in1_3] -> lag10/4(in1_3)
  [in1_4] -> lag10/5(in1_4)
  [in1_5] -> lag10/6(in1_5)
  [in1_6] -> lag10/7(in1_6)
  [in1_7] -> lag10/8(in1_7)
  [in2_10] -> lag10/9(in2_0)
  [in2_11] -> lag10/10(in2_1)
  [in2_12] -> lag10/11(in2_2)
  [in2_13] -> lag10/12(in2_3)
  [in2_14] -> lag10/13(in2_4)
  [in2_15] -> lag10/14(in2_5)
  [in2_16] -> lag10/15(in2_6)
  [in2_17] -> lag10/16(in2_7)
  [reset] -> lag10/17(rst)
  [enable] -> lag10/18(en)
  lag10/1(acc_out) -> out10/1
  [in1_0] -> lag11/1(in1_0)
  [in1_1] -> lag11/2(in1_1)
  [in1_2] -> lag11/3(in1_2)
  [in1_3] -> lag11/4(in1_3)
  [in1_4] -> lag11/5(in1_4)
  [in1_5] -> lag11/6(in1_5)
  [in1_6] -> lag11/7(in1_6)
  [in1_7] -> lag11/8(in1_7)
  [in2_11] -> lag11/9(in2_0)
  [in2_12] -> lag11/10(in2_1)
  [in2_13] -> lag11/11(in2_2)
  [in2_14] -> lag11/12(in2_3)
  [in2_15] -> lag11/13(in2_4)
  [in2_16] -> lag11/14(in2_5)
  [in2_17] -> lag11/15(in2_6)
  [in2_18] -> lag11/16(in2_7)
  [reset] -> lag11/17(rst)
  [enable] -> lag11/18(en)
  lag11/1(acc_out) -> out11/1
  [in1_0] -> lag12/1(in1_0)
  [in1_1] -> lag12/2(in1_1)
  [in1_2] -> lag12/3(in1_2)
  [in1_3] -> lag12/4(in1_3)
  [in1_4] -> lag12/5(in1_4)
  [in1_5] -> lag12/6(in1_5)
  [in1_6] -> lag12/7(in1_6)
  [in1_7] -> lag12/8(in1_7)
  [in2_12] -> lag12/9(in2_0)
  [in2_13] -> lag12/10(in2_1)
  [in2_14] -> lag12/11(in2_2)
  [in2_15] -> lag12/12(in2_3)
  [in2_16] -> lag12/13(in2_4)
  [in2_17] -> lag12/14(in2_5)
  [in2_18] -> lag12/15(in2_6)
  [in2_19] -> lag12/16(in2_7)
  [reset] -> lag12/17(rst)
  [enable] -> lag12/18(en)
  lag12/1(acc_out) -> out12/1
  [in1_0] -> lag13/1(in1_0)
  [in1_1] -> lag13/2(in1_1)
  [in1_2] -> lag13/3(in1_2)
  [in1_3] -> lag13/4(in1_3)
  [in1_4] -> lag13/5(in1_4)
  [in1_5] -> lag13/6(in1_5)
  [in1_6] -> lag13/7(in1_6)
  [in1_7] -> lag13/8(in1_7)
  [in2_13] -> lag13/9(in2_0)
  [in2_14] -> lag13/10(in2_1)
  [in2_15] -> lag13/11(in2_2)
  [in2_16] -> lag13/12(in2_3)
  [in2_17] -> lag13/13(in2_4)
  [in2_18] -> lag13/14(in2_5)
  [in2_19] -> lag13/15(in2_6)
  [in2_20] -> lag13/16(in2_7)
  [reset] -> lag13/17(rst)
  [enable] -> lag13/18(en)
  lag13/1(acc_out) -> out13/1
  [in1_0] -> lag14/1(in1_0)
  [in1_1] -> lag14/2(in1_1)
  [in1_2] -> lag14/3(in1_2)
  [in1_3] -> lag14/4(in1_3)
  [in1_4] -> lag14/5(in1_4)
  [in1_5] -> lag14/6(in1_5)
  [in1_6] -> lag14/7(in1_6)
  [in1_7] -> lag14/8(in1_7)
  [in2_14] -> lag14/9(in2_0)
  [in2_15] -> lag14/10(in2_1)
  [in2_16] -> lag14/11(in2_2)
  [in2_17] -> lag14/12(in2_3)
  [in2_18] -> lag14/13(in2_4)
  [in2_19] -> lag14/14(in2_5)
  [in2_20] -> lag14/15(in2_6)
  [in2_21] -> lag14/16(in2_7)
  [reset] -> lag14/17(rst)
  [enable] -> lag14/18(en)
  lag14/1(acc_out) -> out14/1
  [in1_0] -> lag15/1(in1_0)
  [in1_1] -> lag15/2(in1_1)
  [in1_2] -> lag15/3(in1_2)
  [in1_3] -> lag15/4(in1_3)
  [in1_4] -> lag15/5(in1_4)
  [in1_5] -> lag15/6(in1_5)
  [in1_6] -> lag15/7(in1_6)
  [in1_7] -> lag15/8(in1_7)
  [in2_15] -> lag15/9(in2_0)
  [in2_16] -> lag15/10(in2_1)
  [in2_17] -> lag15/11(in2_2)
  [in2_18] -> lag15/12(in2_3)
  [in2_19] -> lag15/13(in2_4)
  [in2_20] -> lag15/14(in2_5)
  [in2_21] -> lag15/15(in2_6)
  [in2_22] -> lag15/16(in2_7)
  [reset] -> lag15/17(rst)
  [enable] -> lag15/18(en)
  lag15/1(acc_out) -> out15/1

== system bee2_calib_corr/reorder0 ==
  in : Inport  Orientation=up  OutDataType=sfix(16)  OutScaling=2^0
  Concat{1..4} (4 alike) : xbsIndex_r4/Concat  num_inputs=2  dbl_ovrd=off  block_type=concat
      settings: block_version=10.1.3
  Slice18 : xbsIndex_r4/Slice  nbits=8  boolean_output=off  mode=Upper Bit Location + Width  bit1=0  base1=MSB of Input  bit0=0  base0=LSB of Input  dbl_ovrd=off  block_type=slice
      settings: infoedit="Extracts a given range of bits from each input sample and presents it at the output.  The output type is ordinarily unsigned with binary point at zero, but can be Boolean when the slice is one bit wide.<P><P>Hardware notes: In hardware this block costs nothing."  block_version=10.1.3
  Slice19 : xbsIndex_r4/Slice  nbits=8  boolean_output=off  mode=Upper Bit Location + Width  bit1=-8  base1=MSB of Input  bit0=0  base0=LSB of Input  dbl_ovrd=off  block_type=slice
      settings: infoedit="Extracts a given range of bits from each input sample and presents it at the output.  The output type is ordinarily unsigned with binary point at zero, but can be Boolean when the slice is one bit wide.<P><P>Hardware notes: In hardware this block costs nothing."  block_version=10.1.3
  Slice2 : xbsIndex_r4/Slice  nbits=32  boolean_output=off  mode=Upper Bit Location + Width  bit1=0  base1=MSB of Input  bit0=0  base0=LSB of Input  dbl_ovrd=off  block_type=slice
      settings: infoedit="Extracts a given range of bits from each input sample and presents it at the output.  The output type is ordinarily unsigned with binary point at zero, but can be Boolean when the slice is one bit wide.<P><P>Hardware notes: In hardware this block costs nothing."  block_version=10.1.3
  Slice20 : xbsIndex_r4/Slice  nbits=8  boolean_output=off  mode=Upper Bit Location + Width  bit1=-16  base1=MSB of Input  bit0=0  base0=LSB of Input  dbl_ovrd=off  block_type=slice
      settings: infoedit="Extracts a given range of bits from each input sample and presents it at the output.  The output type is ordinarily unsigned with binary point at zero, but can be Boolean when the slice is one bit wide.<P><P>Hardware notes: In hardware this block costs nothing."  block_version=10.1.3
  Slice21 : xbsIndex_r4/Slice  nbits=8  boolean_output=off  mode=Upper Bit Location + Width  bit1=-24  base1=MSB of Input  bit0=0  base0=LSB of Input  dbl_ovrd=off  block_type=slice
      settings: infoedit="Extracts a given range of bits from each input sample and presents it at the output.  The output type is ordinarily unsigned with binary point at zero, but can be Boolean when the slice is one bit wide.<P><P>Hardware notes: In hardware this block costs nothing."  block_version=10.1.3
  Slice22 : xbsIndex_r4/Slice  nbits=8  boolean_output=off  mode=Upper Bit Location + Width  bit1=0  base1=MSB of Input  bit0=0  base0=LSB of Input  dbl_ovrd=off  block_type=slice
      settings: infoedit="Extracts a given range of bits from each input sample and presents it at the output.  The output type is ordinarily unsigned with binary point at zero, but can be Boolean when the slice is one bit wide.<P><P>Hardware notes: In hardware this block costs nothing."  block_version=10.1.3
  Slice23 : xbsIndex_r4/Slice  nbits=8  boolean_output=off  mode=Upper Bit Location + Width  bit1=-8  base1=MSB of Input  bit0=0  base0=LSB of Input  dbl_ovrd=off  block_type=slice
      settings: infoedit="Extracts a given range of bits from each input sample and presents it at the output.  The output type is ordinarily unsigned with binary point at zero, but can be Boolean when the slice is one bit wide.<P><P>Hardware notes: In hardware this block costs nothing."  block_version=10.1.3
  Slice24 : xbsIndex_r4/Slice  nbits=8  boolean_output=off  mode=Upper Bit Location + Width  bit1=-16  base1=MSB of Input  bit0=0  base0=LSB of Input  dbl_ovrd=off  block_type=slice
      settings: infoedit="Extracts a given range of bits from each input sample and presents it at the output.  The output type is ordinarily unsigned with binary point at zero, but can be Boolean when the slice is one bit wide.<P><P>Hardware notes: In hardware this block costs nothing."  block_version=10.1.3
  Slice25 : xbsIndex_r4/Slice  nbits=8  boolean_output=off  mode=Upper Bit Location + Width  bit1=-24  base1=MSB of Input  bit0=0  base0=LSB of Input  dbl_ovrd=off  block_type=slice
      settings: infoedit="Extracts a given range of bits from each input sample and presents it at the output.  The output type is ordinarily unsigned with binary point at zero, but can be Boolean when the slice is one bit wide.<P><P>Hardware notes: In hardware this block costs nothing."  block_version=10.1.3
  Slice3 : xbsIndex_r4/Slice  nbits=32  boolean_output=off  mode=Upper Bit Location + Width  bit1=-32  base1=MSB of Input  bit0=0  base0=LSB of Input  dbl_ovrd=off  block_type=slice
      settings: infoedit="Extracts a given range of bits from each input sample and presents it at the output.  The output type is ordinarily unsigned with binary point at zero, but can be Boolean when the slice is one bit wide.<P><P>Hardware notes: In hardware this block costs nothing."  block_version=10.1.3
  out0 : Outport  OutDataType=sfix(16)  OutScaling=2^0
  out1 : Outport  Port=2  OutDataType=sfix(16)  OutScaling=2^0
  out2 : Outport  Port=3  OutDataType=sfix(16)  OutScaling=2^0
  out3 : Outport  Port=4  OutDataType=sfix(16)  OutScaling=2^0
  wires:
  in/1 -> Slice2/1, Slice3/1
  Slice2/1 -> Slice21/1, Slice20/1, Slice19/1, Slice18/1
  Slice3/1 -> Slice22/1, Slice23/1, Slice24/1, Slice25/1
  Slice18/1 -> Concat3/1
  Slice22/1 -> Concat3/2
  Slice19/1 -> Concat1/1
  Slice23/1 -> Concat1/2
  Slice20/1 -> Concat4/1
  Slice24/1 -> Concat4/2
  Slice21/1 -> Concat2/1
  Slice25/1 -> Concat2/2
  Concat3/1 -> out0/1
  Concat1/1 -> out1/1
  Concat4/1 -> out2/1
  Concat2/1 -> out3/1

== system bee2_calib_corr/reorder1 ==
  in : Inport  Orientation=up  OutDataType=sfix(16)  OutScaling=2^0
  Concat{1..4} (4 alike) : xbsIndex_r4/Concat  num_inputs=2  dbl_ovrd=off  block_type=concat
      settings: block_version=10.1.3
  Slice18 : xbsIndex_r4/Slice  nbits=8  boolean_output=off  mode=Upper Bit Location + Width  bit1=0  base1=MSB of Input  bit0=0  base0=LSB of Input  dbl_ovrd=off  block_type=slice
      settings: infoedit="Extracts a given range of bits from each input sample and presents it at the output.  The output type is ordinarily unsigned with binary point at zero, but can be Boolean when the slice is one bit wide.<P><P>Hardware notes: In hardware this block costs nothing."  block_version=10.1.3
  Slice19 : xbsIndex_r4/Slice  nbits=8  boolean_output=off  mode=Upper Bit Location + Width  bit1=-8  base1=MSB of Input  bit0=0  base0=LSB of Input  dbl_ovrd=off  block_type=slice
      settings: infoedit="Extracts a given range of bits from each input sample and presents it at the output.  The output type is ordinarily unsigned with binary point at zero, but can be Boolean when the slice is one bit wide.<P><P>Hardware notes: In hardware this block costs nothing."  block_version=10.1.3
  Slice2 : xbsIndex_r4/Slice  nbits=32  boolean_output=off  mode=Upper Bit Location + Width  bit1=0  base1=MSB of Input  bit0=0  base0=LSB of Input  dbl_ovrd=off  block_type=slice
      settings: infoedit="Extracts a given range of bits from each input sample and presents it at the output.  The output type is ordinarily unsigned with binary point at zero, but can be Boolean when the slice is one bit wide.<P><P>Hardware notes: In hardware this block costs nothing."  block_version=10.1.3
  Slice20 : xbsIndex_r4/Slice  nbits=8  boolean_output=off  mode=Upper Bit Location + Width  bit1=-16  base1=MSB of Input  bit0=0  base0=LSB of Input  dbl_ovrd=off  block_type=slice
      settings: infoedit="Extracts a given range of bits from each input sample and presents it at the output.  The output type is ordinarily unsigned with binary point at zero, but can be Boolean when the slice is one bit wide.<P><P>Hardware notes: In hardware this block costs nothing."  block_version=10.1.3
  Slice21 : xbsIndex_r4/Slice  nbits=8  boolean_output=off  mode=Upper Bit Location + Width  bit1=-24  base1=MSB of Input  bit0=0  base0=LSB of Input  dbl_ovrd=off  block_type=slice
      settings: infoedit="Extracts a given range of bits from each input sample and presents it at the output.  The output type is ordinarily unsigned with binary point at zero, but can be Boolean when the slice is one bit wide.<P><P>Hardware notes: In hardware this block costs nothing."  block_version=10.1.3
  Slice22 : xbsIndex_r4/Slice  nbits=8  boolean_output=off  mode=Upper Bit Location + Width  bit1=0  base1=MSB of Input  bit0=0  base0=LSB of Input  dbl_ovrd=off  block_type=slice
      settings: infoedit="Extracts a given range of bits from each input sample and presents it at the output.  The output type is ordinarily unsigned with binary point at zero, but can be Boolean when the slice is one bit wide.<P><P>Hardware notes: In hardware this block costs nothing."  block_version=10.1.3
  Slice23 : xbsIndex_r4/Slice  nbits=8  boolean_output=off  mode=Upper Bit Location + Width  bit1=-8  base1=MSB of Input  bit0=0  base0=LSB of Input  dbl_ovrd=off  block_type=slice
      settings: infoedit="Extracts a given range of bits from each input sample and presents it at the output.  The output type is ordinarily unsigned with binary point at zero, but can be Boolean when the slice is one bit wide.<P><P>Hardware notes: In hardware this block costs nothing."  block_version=10.1.3
  Slice24 : xbsIndex_r4/Slice  nbits=8  boolean_output=off  mode=Upper Bit Location + Width  bit1=-16  base1=MSB of Input  bit0=0  base0=LSB of Input  dbl_ovrd=off  block_type=slice
      settings: infoedit="Extracts a given range of bits from each input sample and presents it at the output.  The output type is ordinarily unsigned with binary point at zero, but can be Boolean when the slice is one bit wide.<P><P>Hardware notes: In hardware this block costs nothing."  block_version=10.1.3
  Slice25 : xbsIndex_r4/Slice  nbits=8  boolean_output=off  mode=Upper Bit Location + Width  bit1=-24  base1=MSB of Input  bit0=0  base0=LSB of Input  dbl_ovrd=off  block_type=slice
      settings: infoedit="Extracts a given range of bits from each input sample and presents it at the output.  The output type is ordinarily unsigned with binary point at zero, but can be Boolean when the slice is one bit wide.<P><P>Hardware notes: In hardware this block costs nothing."  block_version=10.1.3
  Slice3 : xbsIndex_r4/Slice  nbits=32  boolean_output=off  mode=Upper Bit Location + Width  bit1=-32  base1=MSB of Input  bit0=0  base0=LSB of Input  dbl_ovrd=off  block_type=slice
      settings: infoedit="Extracts a given range of bits from each input sample and presents it at the output.  The output type is ordinarily unsigned with binary point at zero, but can be Boolean when the slice is one bit wide.<P><P>Hardware notes: In hardware this block costs nothing."  block_version=10.1.3
  out0 : Outport  OutDataType=sfix(16)  OutScaling=2^0
  out1 : Outport  Port=2  OutDataType=sfix(16)  OutScaling=2^0
  out2 : Outport  Port=3  OutDataType=sfix(16)  OutScaling=2^0
  out3 : Outport  Port=4  OutDataType=sfix(16)  OutScaling=2^0
  wires:
  Concat2/1 -> out3/1
  Concat4/1 -> out2/1
  Concat1/1 -> out1/1
  Concat3/1 -> out0/1
  Slice25/1 -> Concat2/2
  Slice21/1 -> Concat2/1
  Slice24/1 -> Concat4/2
  Slice20/1 -> Concat4/1
  Slice23/1 -> Concat1/2
  Slice19/1 -> Concat1/1
  Slice22/1 -> Concat3/2
  Slice18/1 -> Concat3/1
  Slice3/1 -> Slice25/1, Slice24/1, Slice23/1, Slice22/1
  Slice2/1 -> Slice18/1, Slice19/1, Slice20/1, Slice21/1
  in/1 -> Slice3/1, Slice2/1
